simulink model "VirtualADAPT"  (Simulink 8.9)
settings: Solver=ode45  StartTime=0.0  StopTime=inf  RelTol=1e-3  EnableMultiTasking=on
PostLoadFcn:
  | FaultInjectionGUIHandle = FaultInjectionGUI;
InitFcn:
  | VirtualADAPTDataStructure
undefined symbols (not defined in the file or in its folder): ln
libraries: simulink x363
(part 25 of 26)

== system VirtualADAPT/VirtualADAPTv1/PowerDistribution/ACLoadBank1/L1A/LGT6/LightBulb2/R/Computing R ==
  In1 : Inport
  RNominal : Inport  Port=2
  Fault Profile : Inport  Port=3
  Step : Inport  Port=4
  Ramp : Inport  Port=5
  JuncDB : Inport  Port=6
  Bond : Inport  Port=7
  Junction Type : Inport  Port=8
  CalcCausality : Fcn  Expr=(u(1) == u(2) && u(3) ==1) || (u(1) !=u(2) && u(3) == 0)
  CalcOutput : Fcn  Expr=(u(1)*u(2)^u(3))
  MinusOne : Constant  Value=-1
  Mux1 : Mux  Inputs=3
  Mux2 : Mux  Inputs=3
  One : Constant
  Switch1 : MultiPortSwitch  SaturateOnIntegerOverflow=on  InputSameDT=on
  Switch2 : MultiPortSwitch  DataPortOrder=Zero-based contiguous  Inputs=2  zeroidx=on  SaturateOnIntegerOverflow=on  InputSameDT=on
  Out1 : Outport
  Out2 : Outport  Port=2
  wires:
  In1/1 -> Mux2/1
  Fault Profile/1 -> Switch1/1
  RNominal/1 -> Switch1/2
  Step/1 -> Switch1/3
  Ramp/1 -> Switch1/4
  Switch1/1 -> Out2/1, Mux2/2
  JuncDB/1 -> Mux1/1
  Bond/1 -> Mux1/2
  Junction Type/1 -> Mux1/3
  Mux1/1 -> CalcCausality/1
  CalcCausality/1 -> Switch2/1
  One/1 -> Switch2/2
  MinusOne/1 -> Switch2/3
  Switch2/1 -> Mux2/3
  Mux2/1 -> CalcOutput/1
  CalcOutput/1 -> Out1/1

== system VirtualADAPT/VirtualADAPTv1/PowerDistribution/ACLoadBank1/L1A/LGT6/LightBulb2/TemperatureModel/AmbTemp ==
  F : Inport
  Se : Inport  Port=2
  Bias : Step  Time=FT  After=FM  SampleTime=0
  Fault Inject : Subsystem
  Fault Profile : Constant  Value=FP
  Mult2 : Product  SaturateOnIntegerOverflow=on  RndMeth=Zero  InputSameDT=on  OutDataTypeStr=Inherit: Same as first input
  Multiply : Product  SaturateOnIntegerOverflow=on  RndMeth=Zero  InputSameDT=on  OutDataTypeStr=Inherit: Same as first input
  Ramp : simulink/Sources/Ramp  slope=FM  start=FT  X0=0
  Step : Step  Time=FT  Before=1  After=(1 + FM)  SampleTime=0
  StuckAt : Step  Time=FT  Before=1  After=0  SampleTime=0
  StuckAt2 : Step  Time=FT  After=FM  SampleTime=0
  Sum : Sum  SaturateOnIntegerOverflow=on  InputSameDT=on  OutDataTypeStr=Inherit: Same as first input
  Sum2 : Sum  SaturateOnIntegerOverflow=on  InputSameDT=on  OutDataTypeStr=Inherit: Same as first input
  Sum3 : Sum  SaturateOnIntegerOverflow=on  InputSameDT=on  OutDataTypeStr=Inherit: Same as first input
  E : Outport
  wires:
  Fault Inject/1(Out1) -> E/1
  Se/1 -> Fault Inject/1(Nominal), Multiply/1, Sum/1, Sum2/1, Mult2/1
  Fault Profile/1 -> Fault Inject/2(Fault Profile)
  Step/1 -> Multiply/2
  Ramp/1 -> Sum/2
  Multiply/1 -> Fault Inject/3(Step)
  Sum/1 -> Fault Inject/4(Ramp)
  Bias/1 -> Sum2/2
  Sum2/1 -> Fault Inject/5(Bias)
  StuckAt/1 -> Mult2/2
  Mult2/1 -> Sum3/1
  StuckAt2/1 -> Sum3/2
  Sum3/1 -> Fault Inject/6(stuckAt)

== system VirtualADAPT/VirtualADAPTv1/PowerDistribution/ACLoadBank1/L1A/LGT6/LightBulb2/TemperatureModel/Ctheta ==
  F : Inport
  Capacitance : Constant  Value=C
  Data Type Conversion : DataTypeConversion  OutDataTypeStr=boolean
  Detect Change : simulink/Logic and Bit Operations/Detect Change  vinit=0  InputProcessing=Inherited  OutDataTypeStr=uint8
  Fault Inject : Subsystem
  Fault Profile : Constant  Value=FP
  InitialCondition : Constant  Value=IC
  Integrator : Integrator  ExternalReset=either  InitialConditionSource=external
  Product1 : Product  SaturateOnIntegerOverflow=on  RndMeth=Zero  InputSameDT=on  OutDataTypeStr=Inherit: Same as first input
  Product2 : Product  SaturateOnIntegerOverflow=on  RndMeth=Zero  InputSameDT=on  OutDataTypeStr=Inherit: Same as first input
  Ramp : simulink/Sources/Ramp  slope=FM  start=FT  X0=C
  Reciprocal : Math  Operator=reciprocal  SaturateOnIntegerOverflow=on
  Step : Step  Time=FT  Before=C  After=(1 + FM)*C  SampleTime=0
  E : Outport
  C : Outport  Port=2
  q : Outport  Port=3
  wires:
  F/1 -> Integrator/1
  InitialCondition/1 -> Product1/1, Detect Change/1
  Capacitance/1 -> Fault Inject/1(Nominal), Product1/2
  Product1/1 -> Integrator/3
  Integrator/1 -> Product2/1, q/1
  Fault Inject/1(Out1) -> Reciprocal/1, C/1
  Reciprocal/1 -> Product2/2
  Fault Profile/1 -> Fault Inject/2(Fault Profile)
  Step/1 -> Fault Inject/3(Step)
  Ramp/1 -> Fault Inject/4(Ramp)
  Product2/1 -> E/1
  Detect Change/1 -> Data Type Conversion/1
  Data Type Conversion/1 -> Integrator/2

== system VirtualADAPT/VirtualADAPTv1/PowerDistribution/ACLoadBank1/L1A/LGT6/LightBulb2/TemperatureModel/ModAmbTemp ==
  u(1) : Inport
  Function : Fcn  Expr=-u(1)
  Mux : Mux  Inputs=1
  y(1) : Outport
  wires:
  u(1)/1 -> Mux/1
  Mux/1 -> Function/1
  Function/1 -> y(1)/1

== system VirtualADAPT/VirtualADAPTv1/PowerDistribution/ACLoadBank1/L1A/LGT6/LightBulb2/TemperatureModel/ModPs ==
  u(1) : Inport
  Function : Fcn  Expr=u(1)
  Mux : Mux  Inputs=1
  y(1) : Outport
  wires:
  u(1)/1 -> Mux/1
  Mux/1 -> Function/1
  Function/1 -> y(1)/1

== system VirtualADAPT/VirtualADAPTv1/PowerDistribution/ACLoadBank1/L1A/LGT6/LightBulb2/TemperatureModel/OJ ==
  u(1) : Inport
  u(2) : Inport  Port=2
  u(3) : Inport  Port=3
  Adder : Sum  SaturateOnIntegerOverflow=on  InputSameDT=on  OutDataTypeStr=Inherit: Same as first input
  Gain : Gain  SaturateOnIntegerOverflow=on  OutDataTypeStr=Inherit: Same as input  ParamDataTypeStr=Inherit: Same as input
  y(1) : Outport
  y(2) : Outport  Port=2
  y(3) : Outport  Port=3
  O : Outport  Port=4
  wires:
  Adder/1 -> Gain/1
  u(2)/1 -> y(1)/1, y(3)/1, O/1
  u(1)/1 -> Adder/1
  Gain/1 -> y(2)/1
  u(3)/1 -> Adder/2

== system VirtualADAPT/VirtualADAPTv1/PowerDistribution/ACLoadBank1/L1A/LGT6/LightBulb2/TemperatureModel/Rtheta ==
  In : Inport
  Bond : Constant  Value=2
  Computing R : Subsystem
  Fault Profile : Constant  Value=FP
  JuncDB : Constant  Value=2
  Junction Type : Constant
  Ramp : simulink/Sources/Ramp  slope=FM  start=FT  X0=R
  Resistor Value : Constant  Value=R
  Step : Step  Time=FT  Before=R  After=(1 + FM)*R  SampleTime=0
  Out : Outport
  R : Outport  Port=2
  wires:
  Computing R/1(Out1) -> Out/1
  In/1 -> Computing R/1(In1)
  Resistor Value/1 -> Computing R/2(RNominal)
  Fault Profile/1 -> Computing R/3(Fault Profile)
  Step/1 -> Computing R/4(Step)
  Ramp/1 -> Computing R/5(Ramp)
  JuncDB/1 -> Computing R/6(JuncDB)
  Bond/1 -> Computing R/7(Bond)
  Junction Type/1 -> Computing R/8(Junction Type)
  Computing R/2(Out2) -> R/1

== system VirtualADAPT/VirtualADAPTv1/PowerDistribution/ACLoadBank1/L1A/LGT6/LightBulb2/TemperatureModel/SfPs ==
  E : Inport
  Sf : Inport  Port=2
  Bias : Step  Time=FT  After=FM  SampleTime=0
  Fault Inject : Subsystem
  Fault Profile : Constant  Value=FP
  Mult2 : Product  SaturateOnIntegerOverflow=on  RndMeth=Zero  InputSameDT=on  OutDataTypeStr=Inherit: Same as first input
  Multiply : Product  SaturateOnIntegerOverflow=on  RndMeth=Zero  InputSameDT=on  OutDataTypeStr=Inherit: Same as first input
  Ramp : simulink/Sources/Ramp  slope=FM  start=FT  X0=0
  Step : Step  Time=FT  Before=1  After=(1 + FM)  SampleTime=0
  StuckAt : Step  Time=FT  Before=1  After=0  SampleTime=0
  StuckAt2 : Step  Time=FT  After=FM  SampleTime=0
  Sum : Sum  SaturateOnIntegerOverflow=on  InputSameDT=on  OutDataTypeStr=Inherit: Same as first input
  Sum2 : Sum  SaturateOnIntegerOverflow=on  InputSameDT=on  OutDataTypeStr=Inherit: Same as first input
  Sum3 : Sum  SaturateOnIntegerOverflow=on  InputSameDT=on  OutDataTypeStr=Inherit: Same as first input
  F : Outport
  wires:
  Fault Inject/1(Out1) -> F/1
  Sf/1 -> Fault Inject/1(Nominal), Multiply/1, Sum/1, Sum2/1, Mult2/1
  Fault Profile/1 -> Fault Inject/2(Fault Profile)
  Step/1 -> Multiply/2
  Ramp/1 -> Sum/2
  Multiply/1 -> Fault Inject/3(Step)
  Sum/1 -> Fault Inject/4(Ramp)
  Bias/1 -> Sum2/2
  Sum2/1 -> Fault Inject/5(Bias)
  StuckAt/1 -> Mult2/2
  Mult2/1 -> Sum3/1
  StuckAt2/1 -> Sum3/2
  Sum3/1 -> Fault Inject/6(stuckAt)

== system VirtualADAPT/VirtualADAPTv1/PowerDistribution/ACLoadBank1/L1A/LGT6/LightBulb2/TemperatureModel/theta ==
  u(1) : Inport
  u(2) : Inport  Port=2
  u(3) : Inport  Port=3
  Adder : Sum  Inputs=+-  SaturateOnIntegerOverflow=on  InputSameDT=on  OutDataTypeStr=Inherit: Same as first input
  Gain : Gain  SaturateOnIntegerOverflow=on  OutDataTypeStr=Inherit: Same as input  ParamDataTypeStr=Inherit: Same as input
  y(1) : Outport
  y(2) : Outport  Port=2
  y(3) : Outport  Port=3
  O : Outport  Port=4
  wires:
  Adder/1 -> Gain/1
  u(3)/1 -> y(1)/1, y(2)/1, O/1
  u(1)/1 -> Adder/1
  u(2)/1 -> Adder/2
  Gain/1 -> y(3)/1

== system VirtualADAPT/VirtualADAPTv1/PowerDistribution/ACLoadBank1/L1A/LGT6/LightBulb3/R/Computing R ==
  In1 : Inport
  RNominal : Inport  Port=2
  Fault Profile : Inport  Port=3
  Step : Inport  Port=4
  Ramp : Inport  Port=5
  JuncDB : Inport  Port=6
  Bond : Inport  Port=7
  Junction Type : Inport  Port=8
  CalcCausality : Fcn  Expr=(u(1) == u(2) && u(3) ==1) || (u(1) !=u(2) && u(3) == 0)
  CalcOutput : Fcn  Expr=(u(1)*u(2)^u(3))
  MinusOne : Constant  Value=-1
  Mux1 : Mux  Inputs=3
  Mux2 : Mux  Inputs=3
  One : Constant
  Switch1 : MultiPortSwitch  SaturateOnIntegerOverflow=on  InputSameDT=on
  Switch2 : MultiPortSwitch  DataPortOrder=Zero-based contiguous  Inputs=2  zeroidx=on  SaturateOnIntegerOverflow=on  InputSameDT=on
  Out1 : Outport
  Out2 : Outport  Port=2
  wires:
  In1/1 -> Mux2/1
  Fault Profile/1 -> Switch1/1
  RNominal/1 -> Switch1/2
  Step/1 -> Switch1/3
  Ramp/1 -> Switch1/4
  Switch1/1 -> Out2/1, Mux2/2
  JuncDB/1 -> Mux1/1
  Bond/1 -> Mux1/2
  Junction Type/1 -> Mux1/3
  Mux1/1 -> CalcCausality/1
  CalcCausality/1 -> Switch2/1
  One/1 -> Switch2/2
  MinusOne/1 -> Switch2/3
  Switch2/1 -> Mux2/3
  Mux2/1 -> CalcOutput/1
  CalcOutput/1 -> Out1/1

== system VirtualADAPT/VirtualADAPTv1/PowerDistribution/ACLoadBank1/L1A/LGT6/LightBulb3/TemperatureModel/AmbTemp ==
  F : Inport
  Se : Inport  Port=2
  Bias : Step  Time=FT  After=FM  SampleTime=0
  Fault Inject : Subsystem
  Fault Profile : Constant  Value=FP
  Mult2 : Product  SaturateOnIntegerOverflow=on  RndMeth=Zero  InputSameDT=on  OutDataTypeStr=Inherit: Same as first input
  Multiply : Product  SaturateOnIntegerOverflow=on  RndMeth=Zero  InputSameDT=on  OutDataTypeStr=Inherit: Same as first input
  Ramp : simulink/Sources/Ramp  slope=FM  start=FT  X0=0
  Step : Step  Time=FT  Before=1  After=(1 + FM)  SampleTime=0
  StuckAt : Step  Time=FT  Before=1  After=0  SampleTime=0
  StuckAt2 : Step  Time=FT  After=FM  SampleTime=0
  Sum : Sum  SaturateOnIntegerOverflow=on  InputSameDT=on  OutDataTypeStr=Inherit: Same as first input
  Sum2 : Sum  SaturateOnIntegerOverflow=on  InputSameDT=on  OutDataTypeStr=Inherit: Same as first input
  Sum3 : Sum  SaturateOnIntegerOverflow=on  InputSameDT=on  OutDataTypeStr=Inherit: Same as first input
  E : Outport
  wires:
  Fault Inject/1(Out1) -> E/1
  Se/1 -> Fault Inject/1(Nominal), Multiply/1, Sum/1, Sum2/1, Mult2/1
  Fault Profile/1 -> Fault Inject/2(Fault Profile)
  Step/1 -> Multiply/2
  Ramp/1 -> Sum/2
  Multiply/1 -> Fault Inject/3(Step)
  Sum/1 -> Fault Inject/4(Ramp)
  Bias/1 -> Sum2/2
  Sum2/1 -> Fault Inject/5(Bias)
  StuckAt/1 -> Mult2/2
  Mult2/1 -> Sum3/1
  StuckAt2/1 -> Sum3/2
  Sum3/1 -> Fault Inject/6(stuckAt)

== system VirtualADAPT/VirtualADAPTv1/PowerDistribution/ACLoadBank1/L1A/LGT6/LightBulb3/TemperatureModel/Ctheta ==
  F : Inport
  Capacitance : Constant  Value=C
  Data Type Conversion : DataTypeConversion  OutDataTypeStr=boolean
  Detect Change : simulink/Logic and Bit Operations/Detect Change  vinit=0  InputProcessing=Inherited  OutDataTypeStr=uint8
  Fault Inject : Subsystem
  Fault Profile : Constant  Value=FP
  InitialCondition : Constant  Value=IC
  Integrator : Integrator  ExternalReset=either  InitialConditionSource=external
  Product1 : Product  SaturateOnIntegerOverflow=on  RndMeth=Zero  InputSameDT=on  OutDataTypeStr=Inherit: Same as first input
  Product2 : Product  SaturateOnIntegerOverflow=on  RndMeth=Zero  InputSameDT=on  OutDataTypeStr=Inherit: Same as first input
  Ramp : simulink/Sources/Ramp  slope=FM  start=FT  X0=C
  Reciprocal : Math  Operator=reciprocal  SaturateOnIntegerOverflow=on
  Step : Step  Time=FT  Before=C  After=(1 + FM)*C  SampleTime=0
  E : Outport
  C : Outport  Port=2
  q : Outport  Port=3
  wires:
  F/1 -> Integrator/1
  InitialCondition/1 -> Product1/1, Detect Change/1
  Capacitance/1 -> Fault Inject/1(Nominal), Product1/2
  Product1/1 -> Integrator/3
  Integrator/1 -> Product2/1, q/1
  Fault Inject/1(Out1) -> Reciprocal/1, C/1
  Reciprocal/1 -> Product2/2
  Fault Profile/1 -> Fault Inject/2(Fault Profile)
  Step/1 -> Fault Inject/3(Step)
  Ramp/1 -> Fault Inject/4(Ramp)
  Product2/1 -> E/1
  Detect Change/1 -> Data Type Conversion/1
  Data Type Conversion/1 -> Integrator/2

== system VirtualADAPT/VirtualADAPTv1/PowerDistribution/ACLoadBank1/L1A/LGT6/LightBulb3/TemperatureModel/ModAmbTemp ==
  u(1) : Inport
  Function : Fcn  Expr=-u(1)
  Mux : Mux  Inputs=1
  y(1) : Outport
  wires:
  u(1)/1 -> Mux/1
  Mux/1 -> Function/1
  Function/1 -> y(1)/1

== system VirtualADAPT/VirtualADAPTv1/PowerDistribution/ACLoadBank1/L1A/LGT6/LightBulb3/TemperatureModel/ModPs ==
  u(1) : Inport
  Function : Fcn  Expr=u(1)
  Mux : Mux  Inputs=1
  y(1) : Outport
  wires:
  u(1)/1 -> Mux/1
  Mux/1 -> Function/1
  Function/1 -> y(1)/1

== system VirtualADAPT/VirtualADAPTv1/PowerDistribution/ACLoadBank1/L1A/LGT6/LightBulb3/TemperatureModel/OJ ==
  u(1) : Inport
  u(2) : Inport  Port=2
  u(3) : Inport  Port=3
  Adder : Sum  SaturateOnIntegerOverflow=on  InputSameDT=on  OutDataTypeStr=Inherit: Same as first input
  Gain : Gain  SaturateOnIntegerOverflow=on  OutDataTypeStr=Inherit: Same as input  ParamDataTypeStr=Inherit: Same as input
  y(1) : Outport
  y(2) : Outport  Port=2
  y(3) : Outport  Port=3
  O : Outport  Port=4
  wires:
  Adder/1 -> Gain/1
  u(3)/1 -> y(1)/1, y(2)/1, O/1
  u(1)/1 -> Adder/1
  u(2)/1 -> Adder/2
  Gain/1 -> y(3)/1

== system VirtualADAPT/VirtualADAPTv1/PowerDistribution/ACLoadBank1/L1A/LGT6/LightBulb3/TemperatureModel/Rtheta ==
  In : Inport
  Bond : Constant  Value=3
  Computing R : Subsystem
  Fault Profile : Constant  Value=FP
  JuncDB : Constant  Value=3
  Junction Type : Constant
  Ramp : simulink/Sources/Ramp  slope=FM  start=FT  X0=R
  Resistor Value : Constant  Value=R
  Step : Step  Time=FT  Before=R  After=(1 + FM)*R  SampleTime=0
  Out : Outport
  R : Outport  Port=2
  wires:
  Computing R/1(Out1) -> Out/1
  In/1 -> Computing R/1(In1)
  Resistor Value/1 -> Computing R/2(RNominal)
  Fault Profile/1 -> Computing R/3(Fault Profile)
  Step/1 -> Computing R/4(Step)
  Ramp/1 -> Computing R/5(Ramp)
  JuncDB/1 -> Computing R/6(JuncDB)
  Bond/1 -> Computing R/7(Bond)
  Junction Type/1 -> Computing R/8(Junction Type)
  Computing R/2(Out2) -> R/1

== system VirtualADAPT/VirtualADAPTv1/PowerDistribution/ACLoadBank1/L1A/LGT6/LightBulb3/TemperatureModel/SfPs ==
  E : Inport
  Sf : Inport  Port=2
  Bias : Step  Time=FT  After=FM  SampleTime=0
  Fault Inject : Subsystem
  Fault Profile : Constant  Value=FP
  Mult2 : Product  SaturateOnIntegerOverflow=on  RndMeth=Zero  InputSameDT=on  OutDataTypeStr=Inherit: Same as first input
  Multiply : Product  SaturateOnIntegerOverflow=on  RndMeth=Zero  InputSameDT=on  OutDataTypeStr=Inherit: Same as first input
  Ramp : simulink/Sources/Ramp  slope=FM  start=FT  X0=0
  Step : Step  Time=FT  Before=1  After=(1 + FM)  SampleTime=0
  StuckAt : Step  Time=FT  Before=1  After=0  SampleTime=0
  StuckAt2 : Step  Time=FT  After=FM  SampleTime=0
  Sum : Sum  SaturateOnIntegerOverflow=on  InputSameDT=on  OutDataTypeStr=Inherit: Same as first input
  Sum2 : Sum  SaturateOnIntegerOverflow=on  InputSameDT=on  OutDataTypeStr=Inherit: Same as first input
  Sum3 : Sum  SaturateOnIntegerOverflow=on  InputSameDT=on  OutDataTypeStr=Inherit: Same as first input
  F : Outport
  wires:
  Fault Inject/1(Out1) -> F/1
  Sf/1 -> Fault Inject/1(Nominal), Multiply/1, Sum/1, Sum2/1, Mult2/1
  Fault Profile/1 -> Fault Inject/2(Fault Profile)
  Step/1 -> Multiply/2
  Ramp/1 -> Sum/2
  Multiply/1 -> Fault Inject/3(Step)
  Sum/1 -> Fault Inject/4(Ramp)
  Bias/1 -> Sum2/2
  Sum2/1 -> Fault Inject/5(Bias)
  StuckAt/1 -> Mult2/2
  Mult2/1 -> Sum3/1
  StuckAt2/1 -> Sum3/2
  Sum3/1 -> Fault Inject/6(stuckAt)

== system VirtualADAPT/VirtualADAPTv1/PowerDistribution/ACLoadBank1/L1A/LGT6/LightBulb3/TemperatureModel/theta ==
  u(1) : Inport
  u(2) : Inport  Port=2
  u(3) : Inport  Port=3
  Adder : Sum  Inputs=-+  SaturateOnIntegerOverflow=on  InputSameDT=on  OutDataTypeStr=Inherit: Same as first input
  Gain : Gain  SaturateOnIntegerOverflow=on  OutDataTypeStr=Inherit: Same as input  ParamDataTypeStr=Inherit: Same as input
  y(1) : Outport
  y(2) : Outport  Port=2
  y(3) : Outport  Port=3
  O : Outport  Port=4
  wires:
  Adder/1 -> Gain/1
  Gain/1 -> y(1)/1
  u(1)/1 -> y(2)/1, y(3)/1, O/1
  u(2)/1 -> Adder/1
  u(3)/1 -> Adder/2

== system VirtualADAPT/VirtualADAPTv1/PowerDistribution/ACLoadBank1/L1F/LGT4/TemperatureModel/AmbTemp/Fault Inject ==
  Nominal : Inport
  Fault Profile : Inport  Port=2
  Step : Inport  Port=3
  Ramp : Inport  Port=4
  Bias : Inport  Port=5
  stuckAt : Inport  Port=6
  Switch1 : MultiPortSwitch  Inputs=5  SaturateOnIntegerOverflow=on  InputSameDT=on
  Out1 : Outport
  wires:
  Nominal/1 -> Switch1/2
  Fault Profile/1 -> Switch1/1
  Step/1 -> Switch1/3
  Ramp/1 -> Switch1/4
  Bias/1 -> Switch1/5
  stuckAt/1 -> Switch1/6
  Switch1/1 -> Out1/1

== system VirtualADAPT/VirtualADAPTv1/PowerDistribution/ACLoadBank1/L1F/LGT4/TemperatureModel/Ctheta/Fault Inject ==
  Nominal : Inport
  Fault Profile : Inport  Port=2
  Step : Inport  Port=3
  Ramp : Inport  Port=4
  Bias : Inport  Port=5
  stuckAt : Inport  Port=6
  Switch1 : MultiPortSwitch  Inputs=5  SaturateOnIntegerOverflow=on  InputSameDT=on
  Out1 : Outport
  wires:
  Nominal/1 -> Switch1/2
  Fault Profile/1 -> Switch1/1
  Step/1 -> Switch1/3
  Ramp/1 -> Switch1/4
  Bias/1 -> Switch1/5
  stuckAt/1 -> Switch1/6
  Switch1/1 -> Out1/1

== system VirtualADAPT/VirtualADAPTv1/PowerDistribution/ACLoadBank1/L1F/LGT4/TemperatureModel/Rtheta/Computing R ==
  In1 : Inport
  RNominal : Inport  Port=2
  Fault Profile : Inport  Port=3
  Step : Inport  Port=4
  Ramp : Inport  Port=5
  JuncDB : Inport  Port=6
  Bond : Inport  Port=7
  Junction Type : Inport  Port=8
  CalcCausality : Fcn  Expr=(u(1) == u(2) && u(3) ==1) || (u(1) !=u(2) && u(3) == 0)
  CalcOutput : Fcn  Expr=(u(1)*u(2)^u(3))
  MinusOne : Constant  Value=-1
  Mux1 : Mux  Inputs=3
  Mux2 : Mux  Inputs=3
  One : Constant
  Switch1 : MultiPortSwitch  SaturateOnIntegerOverflow=on  InputSameDT=on
  Switch2 : MultiPortSwitch  DataPortOrder=Zero-based contiguous  Inputs=2  zeroidx=on  SaturateOnIntegerOverflow=on  InputSameDT=on
  Out1 : Outport
  Out2 : Outport  Port=2
  wires:
  In1/1 -> Mux2/1
  Fault Profile/1 -> Switch1/1
  RNominal/1 -> Switch1/2
  Step/1 -> Switch1/3
  Ramp/1 -> Switch1/4
  Switch1/1 -> Out2/1, Mux2/2
  JuncDB/1 -> Mux1/1
  Bond/1 -> Mux1/2
  Junction Type/1 -> Mux1/3
  Mux1/1 -> CalcCausality/1
  CalcCausality/1 -> Switch2/1
  One/1 -> Switch2/2
  MinusOne/1 -> Switch2/3
  Switch2/1 -> Mux2/3
  Mux2/1 -> CalcOutput/1
  CalcOutput/1 -> Out1/1

== system VirtualADAPT/VirtualADAPTv1/PowerDistribution/ACLoadBank1/L1F/LGT4/TemperatureModel/SfPs/Fault Inject ==
  Nominal : Inport
  Fault Profile : Inport  Port=2
  Step : Inport  Port=3
  Ramp : Inport  Port=4
  Bias : Inport  Port=5
  stuckAt : Inport  Port=6
  Switch1 : MultiPortSwitch  Inputs=5  SaturateOnIntegerOverflow=on  InputSameDT=on
  Out1 : Outport
  wires:
  Nominal/1 -> Switch1/2
  Fault Profile/1 -> Switch1/1
  Step/1 -> Switch1/3
  Ramp/1 -> Switch1/4
  Bias/1 -> Switch1/5
  stuckAt/1 -> Switch1/6
  Switch1/1 -> Out1/1

== system VirtualADAPT/VirtualADAPTv1/PowerDistribution/ACLoadBank2/L2B/LGT2/TemperatureModel/AmbTemp/Fault Inject ==
  Nominal : Inport
  Fault Profile : Inport  Port=2
  Step : Inport  Port=3
  Ramp : Inport  Port=4
  Bias : Inport  Port=5
  stuckAt : Inport  Port=6
  Switch1 : MultiPortSwitch  Inputs=5  SaturateOnIntegerOverflow=on  InputSameDT=on
  Out1 : Outport
  wires:
  Nominal/1 -> Switch1/2
  Fault Profile/1 -> Switch1/1
  Step/1 -> Switch1/3
  Ramp/1 -> Switch1/4
  Bias/1 -> Switch1/5
  stuckAt/1 -> Switch1/6
  Switch1/1 -> Out1/1

== system VirtualADAPT/VirtualADAPTv1/PowerDistribution/ACLoadBank2/L2B/LGT2/TemperatureModel/Ctheta/Fault Inject ==
  Nominal : Inport
  Fault Profile : Inport  Port=2
  Step : Inport  Port=3
  Ramp : Inport  Port=4
  Bias : Inport  Port=5
  stuckAt : Inport  Port=6
  Switch1 : MultiPortSwitch  Inputs=5  SaturateOnIntegerOverflow=on  InputSameDT=on
  Out1 : Outport
  wires:
  Nominal/1 -> Switch1/2
  Fault Profile/1 -> Switch1/1
  Step/1 -> Switch1/3
  Ramp/1 -> Switch1/4
  Bias/1 -> Switch1/5
  stuckAt/1 -> Switch1/6
  Switch1/1 -> Out1/1

== system VirtualADAPT/VirtualADAPTv1/PowerDistribution/ACLoadBank2/L2B/LGT2/TemperatureModel/Rtheta/Computing R ==
  In1 : Inport
  RNominal : Inport  Port=2
  Fault Profile : Inport  Port=3
  Step : Inport  Port=4
  Ramp : Inport  Port=5
  JuncDB : Inport  Port=6
  Bond : Inport  Port=7
  Junction Type : Inport  Port=8
  CalcCausality : Fcn  Expr=(u(1) == u(2) && u(3) ==1) || (u(1) !=u(2) && u(3) == 0)
  CalcOutput : Fcn  Expr=(u(1)*u(2)^u(3))
  MinusOne : Constant  Value=-1
  Mux1 : Mux  Inputs=3
  Mux2 : Mux  Inputs=3
  One : Constant
  Switch1 : MultiPortSwitch  SaturateOnIntegerOverflow=on  InputSameDT=on
  Switch2 : MultiPortSwitch  DataPortOrder=Zero-based contiguous  Inputs=2  zeroidx=on  SaturateOnIntegerOverflow=on  InputSameDT=on
  Out1 : Outport
  Out2 : Outport  Port=2
  wires:
  In1/1 -> Mux2/1
  Fault Profile/1 -> Switch1/1
  RNominal/1 -> Switch1/2
  Step/1 -> Switch1/3
  Ramp/1 -> Switch1/4
  Switch1/1 -> Out2/1, Mux2/2
  JuncDB/1 -> Mux1/1
  Bond/1 -> Mux1/2
  Junction Type/1 -> Mux1/3
  Mux1/1 -> CalcCausality/1
  CalcCausality/1 -> Switch2/1
  One/1 -> Switch2/2
  MinusOne/1 -> Switch2/3
  Switch2/1 -> Mux2/3
  Mux2/1 -> CalcOutput/1
  CalcOutput/1 -> Out1/1

== system VirtualADAPT/VirtualADAPTv1/PowerDistribution/ACLoadBank2/L2B/LGT2/TemperatureModel/SfPs/Fault Inject ==
  Nominal : Inport
  Fault Profile : Inport  Port=2
  Step : Inport  Port=3
  Ramp : Inport  Port=4
  Bias : Inport  Port=5
  stuckAt : Inport  Port=6
  Switch1 : MultiPortSwitch  Inputs=5  SaturateOnIntegerOverflow=on  InputSameDT=on
  Out1 : Outport
  wires:
  Nominal/1 -> Switch1/2
  Fault Profile/1 -> Switch1/1
  Step/1 -> Switch1/3
  Ramp/1 -> Switch1/4
  Bias/1 -> Switch1/5
  stuckAt/1 -> Switch1/6
  Switch1/1 -> Out1/1

== system VirtualADAPT/VirtualADAPTv1/PowerDistribution/ACLoadBank2/L2E/LGT7/LightBulb1/R/Computing R ==
  In1 : Inport
  RNominal : Inport  Port=2
  Fault Profile : Inport  Port=3
  Step : Inport  Port=4
  Ramp : Inport  Port=5
  JuncDB : Inport  Port=6
  Bond : Inport  Port=7
  Junction Type : Inport  Port=8
  CalcCausality : Fcn  Expr=(u(1) == u(2) && u(3) ==1) || (u(1) !=u(2) && u(3) == 0)
  CalcOutput : Fcn  Expr=(u(1)*u(2)^u(3))
  MinusOne : Constant  Value=-1
  Mux1 : Mux  Inputs=3
  Mux2 : Mux  Inputs=3
  One : Constant
  Switch1 : MultiPortSwitch  SaturateOnIntegerOverflow=on  InputSameDT=on
  Switch2 : MultiPortSwitch  DataPortOrder=Zero-based contiguous  Inputs=2  zeroidx=on  SaturateOnIntegerOverflow=on  InputSameDT=on
  Out1 : Outport
  Out2 : Outport  Port=2
  wires:
  In1/1 -> Mux2/1
  Fault Profile/1 -> Switch1/1
  RNominal/1 -> Switch1/2
  Step/1 -> Switch1/3
  Ramp/1 -> Switch1/4
  Switch1/1 -> Out2/1, Mux2/2
  JuncDB/1 -> Mux1/1
  Bond/1 -> Mux1/2
  Junction Type/1 -> Mux1/3
  Mux1/1 -> CalcCausality/1
  CalcCausality/1 -> Switch2/1
  One/1 -> Switch2/2
  MinusOne/1 -> Switch2/3
  Switch2/1 -> Mux2/3
  Mux2/1 -> CalcOutput/1
  CalcOutput/1 -> Out1/1

== system VirtualADAPT/VirtualADAPTv1/PowerDistribution/ACLoadBank2/L2E/LGT7/LightBulb1/TemperatureModel/AmbTemp ==
  F : Inport
  Se : Inport  Port=2
  Bias : Step  Time=FT  After=FM  SampleTime=0
  Fault Inject : Subsystem
  Fault Profile : Constant  Value=FP
  Mult2 : Product  SaturateOnIntegerOverflow=on  RndMeth=Zero  InputSameDT=on  OutDataTypeStr=Inherit: Same as first input
  Multiply : Product  SaturateOnIntegerOverflow=on  RndMeth=Zero  InputSameDT=on  OutDataTypeStr=Inherit: Same as first input
  Ramp : simulink/Sources/Ramp  slope=FM  start=FT  X0=0
  Step : Step  Time=FT  Before=1  After=(1 + FM)  SampleTime=0
  StuckAt : Step  Time=FT  Before=1  After=0  SampleTime=0
  StuckAt2 : Step  Time=FT  After=FM  SampleTime=0
  Sum : Sum  SaturateOnIntegerOverflow=on  InputSameDT=on  OutDataTypeStr=Inherit: Same as first input
  Sum2 : Sum  SaturateOnIntegerOverflow=on  InputSameDT=on  OutDataTypeStr=Inherit: Same as first input
  Sum3 : Sum  SaturateOnIntegerOverflow=on  InputSameDT=on  OutDataTypeStr=Inherit: Same as first input
  E : Outport
  wires:
  Fault Inject/1(Out1) -> E/1
  Se/1 -> Fault Inject/1(Nominal), Multiply/1, Sum/1, Sum2/1, Mult2/1
  Fault Profile/1 -> Fault Inject/2(Fault Profile)
  Step/1 -> Multiply/2
  Ramp/1 -> Sum/2
  Multiply/1 -> Fault Inject/3(Step)
  Sum/1 -> Fault Inject/4(Ramp)
  Bias/1 -> Sum2/2
  Sum2/1 -> Fault Inject/5(Bias)
  StuckAt/1 -> Mult2/2
  Mult2/1 -> Sum3/1
  StuckAt2/1 -> Sum3/2
  Sum3/1 -> Fault Inject/6(stuckAt)

== system VirtualADAPT/VirtualADAPTv1/PowerDistribution/ACLoadBank2/L2E/LGT7/LightBulb1/TemperatureModel/Ctheta ==
  F : Inport
  Capacitance : Constant  Value=C
  Data Type Conversion : DataTypeConversion  OutDataTypeStr=boolean
  Detect Change : simulink/Logic and Bit Operations/Detect Change  vinit=0  InputProcessing=Inherited  OutDataTypeStr=uint8
  Fault Inject : Subsystem
  Fault Profile : Constant  Value=FP
  InitialCondition : Constant  Value=IC
  Integrator : Integrator  ExternalReset=either  InitialConditionSource=external
  Product1 : Product  SaturateOnIntegerOverflow=on  RndMeth=Zero  InputSameDT=on  OutDataTypeStr=Inherit: Same as first input
  Product2 : Product  SaturateOnIntegerOverflow=on  RndMeth=Zero  InputSameDT=on  OutDataTypeStr=Inherit: Same as first input
  Ramp : simulink/Sources/Ramp  slope=FM  start=FT  X0=C
  Reciprocal : Math  Operator=reciprocal  SaturateOnIntegerOverflow=on
  Step : Step  Time=FT  Before=C  After=(1 + FM)*C  SampleTime=0
  E : Outport
  C : Outport  Port=2
  q : Outport  Port=3
  wires:
  F/1 -> Integrator/1
  InitialCondition/1 -> Product1/1, Detect Change/1
  Capacitance/1 -> Fault Inject/1(Nominal), Product1/2
  Product1/1 -> Integrator/3
  Integrator/1 -> Product2/1, q/1
  Fault Inject/1(Out1) -> Reciprocal/1, C/1
  Reciprocal/1 -> Product2/2
  Fault Profile/1 -> Fault Inject/2(Fault Profile)
  Step/1 -> Fault Inject/3(Step)
  Ramp/1 -> Fault Inject/4(Ramp)
  Product2/1 -> E/1
  Detect Change/1 -> Data Type Conversion/1
  Data Type Conversion/1 -> Integrator/2

== system VirtualADAPT/VirtualADAPTv1/PowerDistribution/ACLoadBank2/L2E/LGT7/LightBulb1/TemperatureModel/ModAmbTemp ==
  u(1) : Inport
  Function : Fcn  Expr=-u(1)
  Mux : Mux  Inputs=1
  y(1) : Outport
  wires:
  u(1)/1 -> Mux/1
  Mux/1 -> Function/1
  Function/1 -> y(1)/1

== system VirtualADAPT/VirtualADAPTv1/PowerDistribution/ACLoadBank2/L2E/LGT7/LightBulb1/TemperatureModel/ModPs ==
  u(1) : Inport
  Function : Fcn  Expr=u(1)
  Mux : Mux  Inputs=1
  y(1) : Outport
  wires:
  u(1)/1 -> Mux/1
  Mux/1 -> Function/1
  Function/1 -> y(1)/1

== system VirtualADAPT/VirtualADAPTv1/PowerDistribution/ACLoadBank2/L2E/LGT7/LightBulb1/TemperatureModel/OJ ==
  u(1) : Inport
  u(2) : Inport  Port=2
  u(3) : Inport  Port=3
  Adder : Sum  SaturateOnIntegerOverflow=on  InputSameDT=on  OutDataTypeStr=Inherit: Same as first input
  Gain : Gain  SaturateOnIntegerOverflow=on  OutDataTypeStr=Inherit: Same as input  ParamDataTypeStr=Inherit: Same as input
  y(1) : Outport
  y(2) : Outport  Port=2
  y(3) : Outport  Port=3
  O : Outport  Port=4
  wires:
  Adder/1 -> Gain/1
  u(2)/1 -> y(1)/1, y(3)/1, O/1
  u(1)/1 -> Adder/1
  Gain/1 -> y(2)/1
  u(3)/1 -> Adder/2

== system VirtualADAPT/VirtualADAPTv1/PowerDistribution/ACLoadBank2/L2E/LGT7/LightBulb1/TemperatureModel/Rtheta ==
  In : Inport
  Bond : Constant  Value=2
  Computing R : Subsystem
  Fault Profile : Constant  Value=FP
  JuncDB : Constant  Value=2
  Junction Type : Constant
  Ramp : simulink/Sources/Ramp  slope=FM  start=FT  X0=R
  Resistor Value : Constant  Value=R
  Step : Step  Time=FT  Before=R  After=(1 + FM)*R  SampleTime=0
  Out : Outport
  R : Outport  Port=2
  wires:
  Computing R/1(Out1) -> Out/1
  In/1 -> Computing R/1(In1)
  Resistor Value/1 -> Computing R/2(RNominal)
  Fault Profile/1 -> Computing R/3(Fault Profile)
  Step/1 -> Computing R/4(Step)
  Ramp/1 -> Computing R/5(Ramp)
  JuncDB/1 -> Computing R/6(JuncDB)
  Bond/1 -> Computing R/7(Bond)
  Junction Type/1 -> Computing R/8(Junction Type)
  Computing R/2(Out2) -> R/1

== system VirtualADAPT/VirtualADAPTv1/PowerDistribution/ACLoadBank2/L2E/LGT7/LightBulb1/TemperatureModel/SfPs ==
  E : Inport
  Sf : Inport  Port=2
  Bias : Step  Time=FT  After=FM  SampleTime=0
  Fault Inject : Subsystem
  Fault Profile : Constant  Value=FP
  Mult2 : Product  SaturateOnIntegerOverflow=on  RndMeth=Zero  InputSameDT=on  OutDataTypeStr=Inherit: Same as first input
  Multiply : Product  SaturateOnIntegerOverflow=on  RndMeth=Zero  InputSameDT=on  OutDataTypeStr=Inherit: Same as first input
  Ramp : simulink/Sources/Ramp  slope=FM  start=FT  X0=0
  Step : Step  Time=FT  Before=1  After=(1 + FM)  SampleTime=0
  StuckAt : Step  Time=FT  Before=1  After=0  SampleTime=0
  StuckAt2 : Step  Time=FT  After=FM  SampleTime=0
  Sum : Sum  SaturateOnIntegerOverflow=on  InputSameDT=on  OutDataTypeStr=Inherit: Same as first input
  Sum2 : Sum  SaturateOnIntegerOverflow=on  InputSameDT=on  OutDataTypeStr=Inherit: Same as first input
  Sum3 : Sum  SaturateOnIntegerOverflow=on  InputSameDT=on  OutDataTypeStr=Inherit: Same as first input
  F : Outport
  wires:
  Fault Inject/1(Out1) -> F/1
  Sf/1 -> Fault Inject/1(Nominal), Multiply/1, Sum/1, Sum2/1, Mult2/1
  Fault Profile/1 -> Fault Inject/2(Fault Profile)
  Step/1 -> Multiply/2
  Ramp/1 -> Sum/2
  Multiply/1 -> Fault Inject/3(Step)
  Sum/1 -> Fault Inject/4(Ramp)
  Bias/1 -> Sum2/2
  Sum2/1 -> Fault Inject/5(Bias)
  StuckAt/1 -> Mult2/2
  Mult2/1 -> Sum3/1
  StuckAt2/1 -> Sum3/2
  Sum3/1 -> Fault Inject/6(stuckAt)

== system VirtualADAPT/VirtualADAPTv1/PowerDistribution/ACLoadBank2/L2E/LGT7/LightBulb1/TemperatureModel/theta ==
  u(1) : Inport
  u(2) : Inport  Port=2
  u(3) : Inport  Port=3
  Adder : Sum  Inputs=+-  SaturateOnIntegerOverflow=on  InputSameDT=on  OutDataTypeStr=Inherit: Same as first input
  Gain : Gain  SaturateOnIntegerOverflow=on  OutDataTypeStr=Inherit: Same as input  ParamDataTypeStr=Inherit: Same as input
  y(1) : Outport
  y(2) : Outport  Port=2
  y(3) : Outport  Port=3
  O : Outport  Port=4
  wires:
  Adder/1 -> Gain/1
  u(3)/1 -> y(1)/1, y(2)/1, O/1
  u(1)/1 -> Adder/1
  u(2)/1 -> Adder/2
  Gain/1 -> y(3)/1

== system VirtualADAPT/VirtualADAPTv1/PowerDistribution/ACLoadBank2/L2E/LGT7/LightBulb2/R/Computing R ==
  In1 : Inport
  RNominal : Inport  Port=2
  Fault Profile : Inport  Port=3
  Step : Inport  Port=4
  Ramp : Inport  Port=5
  JuncDB : Inport  Port=6
  Bond : Inport  Port=7
  Junction Type : Inport  Port=8
  CalcCausality : Fcn  Expr=(u(1) == u(2) && u(3) ==1) || (u(1) !=u(2) && u(3) == 0)
  CalcOutput : Fcn  Expr=(u(1)*u(2)^u(3))
  MinusOne : Constant  Value=-1
  Mux1 : Mux  Inputs=3
  Mux2 : Mux  Inputs=3
  One : Constant
  Switch1 : MultiPortSwitch  SaturateOnIntegerOverflow=on  InputSameDT=on
  Switch2 : MultiPortSwitch  DataPortOrder=Zero-based contiguous  Inputs=2  zeroidx=on  SaturateOnIntegerOverflow=on  InputSameDT=on
  Out1 : Outport
  Out2 : Outport  Port=2
  wires:
  In1/1 -> Mux2/1
  Fault Profile/1 -> Switch1/1
  RNominal/1 -> Switch1/2
  Step/1 -> Switch1/3
  Ramp/1 -> Switch1/4
  Switch1/1 -> Out2/1, Mux2/2
  JuncDB/1 -> Mux1/1
  Bond/1 -> Mux1/2
  Junction Type/1 -> Mux1/3
  Mux1/1 -> CalcCausality/1
  CalcCausality/1 -> Switch2/1
  One/1 -> Switch2/2
  MinusOne/1 -> Switch2/3
  Switch2/1 -> Mux2/3
  Mux2/1 -> CalcOutput/1
  CalcOutput/1 -> Out1/1

== system VirtualADAPT/VirtualADAPTv1/PowerDistribution/ACLoadBank2/L2E/LGT7/LightBulb2/TemperatureModel/AmbTemp ==
  F : Inport
  Se : Inport  Port=2
  Bias : Step  Time=FT  After=FM  SampleTime=0
  Fault Inject : Subsystem
  Fault Profile : Constant  Value=FP
  Mult2 : Product  SaturateOnIntegerOverflow=on  RndMeth=Zero  InputSameDT=on  OutDataTypeStr=Inherit: Same as first input
  Multiply : Product  SaturateOnIntegerOverflow=on  RndMeth=Zero  InputSameDT=on  OutDataTypeStr=Inherit: Same as first input
  Ramp : simulink/Sources/Ramp  slope=FM  start=FT  X0=0
  Step : Step  Time=FT  Before=1  After=(1 + FM)  SampleTime=0
  StuckAt : Step  Time=FT  Before=1  After=0  SampleTime=0
  StuckAt2 : Step  Time=FT  After=FM  SampleTime=0
  Sum : Sum  SaturateOnIntegerOverflow=on  InputSameDT=on  OutDataTypeStr=Inherit: Same as first input
  Sum2 : Sum  SaturateOnIntegerOverflow=on  InputSameDT=on  OutDataTypeStr=Inherit: Same as first input
  Sum3 : Sum  SaturateOnIntegerOverflow=on  InputSameDT=on  OutDataTypeStr=Inherit: Same as first input
  E : Outport
  wires:
  Fault Inject/1(Out1) -> E/1
  Se/1 -> Fault Inject/1(Nominal), Multiply/1, Sum/1, Sum2/1, Mult2/1
  Fault Profile/1 -> Fault Inject/2(Fault Profile)
  Step/1 -> Multiply/2
  Ramp/1 -> Sum/2
  Multiply/1 -> Fault Inject/3(Step)
  Sum/1 -> Fault Inject/4(Ramp)
  Bias/1 -> Sum2/2
  Sum2/1 -> Fault Inject/5(Bias)
  StuckAt/1 -> Mult2/2
  Mult2/1 -> Sum3/1
  StuckAt2/1 -> Sum3/2
  Sum3/1 -> Fault Inject/6(stuckAt)

== system VirtualADAPT/VirtualADAPTv1/PowerDistribution/ACLoadBank2/L2E/LGT7/LightBulb2/TemperatureModel/Ctheta ==
  F : Inport
  Capacitance : Constant  Value=C
  Data Type Conversion : DataTypeConversion  OutDataTypeStr=boolean
  Detect Change : simulink/Logic and Bit Operations/Detect Change  vinit=0  InputProcessing=Inherited  OutDataTypeStr=uint8
  Fault Inject : Subsystem
  Fault Profile : Constant  Value=FP
  InitialCondition : Constant  Value=IC
  Integrator : Integrator  ExternalReset=either  InitialConditionSource=external
  Product1 : Product  SaturateOnIntegerOverflow=on  RndMeth=Zero  InputSameDT=on  OutDataTypeStr=Inherit: Same as first input
  Product2 : Product  SaturateOnIntegerOverflow=on  RndMeth=Zero  InputSameDT=on  OutDataTypeStr=Inherit: Same as first input
  Ramp : simulink/Sources/Ramp  slope=FM  start=FT  X0=C
  Reciprocal : Math  Operator=reciprocal  SaturateOnIntegerOverflow=on
  Step : Step  Time=FT  Before=C  After=(1 + FM)*C  SampleTime=0
  E : Outport
  C : Outport  Port=2
  q : Outport  Port=3
  wires:
  F/1 -> Integrator/1
  InitialCondition/1 -> Product1/1, Detect Change/1
  Capacitance/1 -> Fault Inject/1(Nominal), Product1/2
  Product1/1 -> Integrator/3
  Integrator/1 -> Product2/1, q/1
  Fault Inject/1(Out1) -> Reciprocal/1, C/1
  Reciprocal/1 -> Product2/2
  Fault Profile/1 -> Fault Inject/2(Fault Profile)
  Step/1 -> Fault Inject/3(Step)
  Ramp/1 -> Fault Inject/4(Ramp)
  Product2/1 -> E/1
  Detect Change/1 -> Data Type Conversion/1
  Data Type Conversion/1 -> Integrator/2

== system VirtualADAPT/VirtualADAPTv1/PowerDistribution/ACLoadBank2/L2E/LGT7/LightBulb2/TemperatureModel/ModAmbTemp ==
  u(1) : Inport
  Function : Fcn  Expr=-u(1)
  Mux : Mux  Inputs=1
  y(1) : Outport
  wires:
  u(1)/1 -> Mux/1
  Mux/1 -> Function/1
  Function/1 -> y(1)/1

== system VirtualADAPT/VirtualADAPTv1/PowerDistribution/ACLoadBank2/L2E/LGT7/LightBulb2/TemperatureModel/ModPs ==
  u(1) : Inport
  Function : Fcn  Expr=u(1)
  Mux : Mux  Inputs=1
  y(1) : Outport
  wires:
  u(1)/1 -> Mux/1
  Mux/1 -> Function/1
  Function/1 -> y(1)/1

== system VirtualADAPT/VirtualADAPTv1/PowerDistribution/ACLoadBank2/L2E/LGT7/LightBulb2/TemperatureModel/OJ ==
  u(1) : Inport
  u(2) : Inport  Port=2
  u(3) : Inport  Port=3
  Adder : Sum  SaturateOnIntegerOverflow=on  InputSameDT=on  OutDataTypeStr=Inherit: Same as first input
  Gain : Gain  SaturateOnIntegerOverflow=on  OutDataTypeStr=Inherit: Same as input  ParamDataTypeStr=Inherit: Same as input
  y(1) : Outport
  y(2) : Outport  Port=2
  y(3) : Outport  Port=3
  O : Outport  Port=4
  wires:
  Adder/1 -> Gain/1
  u(2)/1 -> y(1)/1, y(3)/1, O/1
  u(1)/1 -> Adder/1
  Gain/1 -> y(2)/1
  u(3)/1 -> Adder/2

== system VirtualADAPT/VirtualADAPTv1/PowerDistribution/ACLoadBank2/L2E/LGT7/LightBulb2/TemperatureModel/Rtheta ==
  In : Inport
  Bond : Constant  Value=2
  Computing R : Subsystem
  Fault Profile : Constant  Value=FP
  JuncDB : Constant  Value=2
  Junction Type : Constant
  Ramp : simulink/Sources/Ramp  slope=FM  start=FT  X0=R
  Resistor Value : Constant  Value=R
  Step : Step  Time=FT  Before=R  After=(1 + FM)*R  SampleTime=0
  Out : Outport
  R : Outport  Port=2
  wires:
  Computing R/1(Out1) -> Out/1
  In/1 -> Computing R/1(In1)
  Resistor Value/1 -> Computing R/2(RNominal)
  Fault Profile/1 -> Computing R/3(Fault Profile)
  Step/1 -> Computing R/4(Step)
  Ramp/1 -> Computing R/5(Ramp)
  JuncDB/1 -> Computing R/6(JuncDB)
  Bond/1 -> Computing R/7(Bond)
  Junction Type/1 -> Computing R/8(Junction Type)
  Computing R/2(Out2) -> R/1

== system VirtualADAPT/VirtualADAPTv1/PowerDistribution/ACLoadBank2/L2E/LGT7/LightBulb2/TemperatureModel/SfPs ==
  E : Inport
  Sf : Inport  Port=2
  Bias : Step  Time=FT  After=FM  SampleTime=0
  Fault Inject : Subsystem
  Fault Profile : Constant  Value=FP
  Mult2 : Product  SaturateOnIntegerOverflow=on  RndMeth=Zero  InputSameDT=on  OutDataTypeStr=Inherit: Same as first input
  Multiply : Product  SaturateOnIntegerOverflow=on  RndMeth=Zero  InputSameDT=on  OutDataTypeStr=Inherit: Same as first input
  Ramp : simulink/Sources/Ramp  slope=FM  start=FT  X0=0
  Step : Step  Time=FT  Before=1  After=(1 + FM)  SampleTime=0
  StuckAt : Step  Time=FT  Before=1  After=0  SampleTime=0
  StuckAt2 : Step  Time=FT  After=FM  SampleTime=0
  Sum : Sum  SaturateOnIntegerOverflow=on  InputSameDT=on  OutDataTypeStr=Inherit: Same as first input
  Sum2 : Sum  SaturateOnIntegerOverflow=on  InputSameDT=on  OutDataTypeStr=Inherit: Same as first input
  Sum3 : Sum  SaturateOnIntegerOverflow=on  InputSameDT=on  OutDataTypeStr=Inherit: Same as first input
  F : Outport
  wires:
  Fault Inject/1(Out1) -> F/1
  Sf/1 -> Fault Inject/1(Nominal), Multiply/1, Sum/1, Sum2/1, Mult2/1
  Fault Profile/1 -> Fault Inject/2(Fault Profile)
  Step/1 -> Multiply/2
  Ramp/1 -> Sum/2
  Multiply/1 -> Fault Inject/3(Step)
  Sum/1 -> Fault Inject/4(Ramp)
  Bias/1 -> Sum2/2
  Sum2/1 -> Fault Inject/5(Bias)
  StuckAt/1 -> Mult2/2
  Mult2/1 -> Sum3/1
  StuckAt2/1 -> Sum3/2
  Sum3/1 -> Fault Inject/6(stuckAt)

== system VirtualADAPT/VirtualADAPTv1/PowerDistribution/ACLoadBank2/L2E/LGT7/LightBulb2/TemperatureModel/theta ==
  u(1) : Inport
  u(2) : Inport  Port=2
  u(3) : Inport  Port=3
  Adder : Sum  Inputs=+-  SaturateOnIntegerOverflow=on  InputSameDT=on  OutDataTypeStr=Inherit: Same as first input
  Gain : Gain  SaturateOnIntegerOverflow=on  OutDataTypeStr=Inherit: Same as input  ParamDataTypeStr=Inherit: Same as input
  y(1) : Outport
  y(2) : Outport  Port=2
  y(3) : Outport  Port=3
  O : Outport  Port=4
  wires:
  Adder/1 -> Gain/1
  u(3)/1 -> y(1)/1, y(2)/1, O/1
  u(1)/1 -> Adder/1
  u(2)/1 -> Adder/2
  Gain/1 -> y(3)/1

== system VirtualADAPT/VirtualADAPTv1/PowerDistribution/ACLoadBank2/L2E/LGT7/LightBulb3/R/Computing R ==
  In1 : Inport
  RNominal : Inport  Port=2
  Fault Profile : Inport  Port=3
  Step : Inport  Port=4
  Ramp : Inport  Port=5
  JuncDB : Inport  Port=6
  Bond : Inport  Port=7
  Junction Type : Inport  Port=8
  CalcCausality : Fcn  Expr=(u(1) == u(2) && u(3) ==1) || (u(1) !=u(2) && u(3) == 0)
  CalcOutput : Fcn  Expr=(u(1)*u(2)^u(3))
  MinusOne : Constant  Value=-1
  Mux1 : Mux  Inputs=3
  Mux2 : Mux  Inputs=3
  One : Constant
  Switch1 : MultiPortSwitch  SaturateOnIntegerOverflow=on  InputSameDT=on
  Switch2 : MultiPortSwitch  DataPortOrder=Zero-based contiguous  Inputs=2  zeroidx=on  SaturateOnIntegerOverflow=on  InputSameDT=on
  Out1 : Outport
  Out2 : Outport  Port=2
  wires:
  In1/1 -> Mux2/1
  Fault Profile/1 -> Switch1/1
  RNominal/1 -> Switch1/2
  Step/1 -> Switch1/3
  Ramp/1 -> Switch1/4
  Switch1/1 -> Out2/1, Mux2/2
  JuncDB/1 -> Mux1/1
  Bond/1 -> Mux1/2
  Junction Type/1 -> Mux1/3
  Mux1/1 -> CalcCausality/1
  CalcCausality/1 -> Switch2/1
  One/1 -> Switch2/2
  MinusOne/1 -> Switch2/3
  Switch2/1 -> Mux2/3
  Mux2/1 -> CalcOutput/1
  CalcOutput/1 -> Out1/1

== system VirtualADAPT/VirtualADAPTv1/PowerDistribution/ACLoadBank2/L2E/LGT7/LightBulb3/TemperatureModel/AmbTemp ==
  F : Inport
  Se : Inport  Port=2
  Bias : Step  Time=FT  After=FM  SampleTime=0
  Fault Inject : Subsystem
  Fault Profile : Constant  Value=FP
  Mult2 : Product  SaturateOnIntegerOverflow=on  RndMeth=Zero  InputSameDT=on  OutDataTypeStr=Inherit: Same as first input
  Multiply : Product  SaturateOnIntegerOverflow=on  RndMeth=Zero  InputSameDT=on  OutDataTypeStr=Inherit: Same as first input
  Ramp : simulink/Sources/Ramp  slope=FM  start=FT  X0=0
  Step : Step  Time=FT  Before=1  After=(1 + FM)  SampleTime=0
  StuckAt : Step  Time=FT  Before=1  After=0  SampleTime=0
  StuckAt2 : Step  Time=FT  After=FM  SampleTime=0
  Sum : Sum  SaturateOnIntegerOverflow=on  InputSameDT=on  OutDataTypeStr=Inherit: Same as first input
  Sum2 : Sum  SaturateOnIntegerOverflow=on  InputSameDT=on  OutDataTypeStr=Inherit: Same as first input
  Sum3 : Sum  SaturateOnIntegerOverflow=on  InputSameDT=on  OutDataTypeStr=Inherit: Same as first input
  E : Outport
  wires:
  Fault Inject/1(Out1) -> E/1
  Se/1 -> Fault Inject/1(Nominal), Multiply/1, Sum/1, Sum2/1, Mult2/1
  Fault Profile/1 -> Fault Inject/2(Fault Profile)
  Step/1 -> Multiply/2
  Ramp/1 -> Sum/2
  Multiply/1 -> Fault Inject/3(Step)
  Sum/1 -> Fault Inject/4(Ramp)
  Bias/1 -> Sum2/2
  Sum2/1 -> Fault Inject/5(Bias)
  StuckAt/1 -> Mult2/2
  Mult2/1 -> Sum3/1
  StuckAt2/1 -> Sum3/2
  Sum3/1 -> Fault Inject/6(stuckAt)

== system VirtualADAPT/VirtualADAPTv1/PowerDistribution/ACLoadBank2/L2E/LGT7/LightBulb3/TemperatureModel/Ctheta ==
  F : Inport
  Capacitance : Constant  Value=C
  Data Type Conversion : DataTypeConversion  OutDataTypeStr=boolean
  Detect Change : simulink/Logic and Bit Operations/Detect Change  vinit=0  InputProcessing=Inherited  OutDataTypeStr=uint8
  Fault Inject : Subsystem
  Fault Profile : Constant  Value=FP
  InitialCondition : Constant  Value=IC
  Integrator : Integrator  ExternalReset=either  InitialConditionSource=external
  Product1 : Product  SaturateOnIntegerOverflow=on  RndMeth=Zero  InputSameDT=on  OutDataTypeStr=Inherit: Same as first input
  Product2 : Product  SaturateOnIntegerOverflow=on  RndMeth=Zero  InputSameDT=on  OutDataTypeStr=Inherit: Same as first input
  Ramp : simulink/Sources/Ramp  slope=FM  start=FT  X0=C
  Reciprocal : Math  Operator=reciprocal  SaturateOnIntegerOverflow=on
  Step : Step  Time=FT  Before=C  After=(1 + FM)*C  SampleTime=0
  E : Outport
  C : Outport  Port=2
  q : Outport  Port=3
  wires:
  F/1 -> Integrator/1
  InitialCondition/1 -> Product1/1, Detect Change/1
  Capacitance/1 -> Fault Inject/1(Nominal), Product1/2
  Product1/1 -> Integrator/3
  Integrator/1 -> Product2/1, q/1
  Fault Inject/1(Out1) -> Reciprocal/1, C/1
  Reciprocal/1 -> Product2/2
  Fault Profile/1 -> Fault Inject/2(Fault Profile)
  Step/1 -> Fault Inject/3(Step)
  Ramp/1 -> Fault Inject/4(Ramp)
  Product2/1 -> E/1
  Detect Change/1 -> Data Type Conversion/1
  Data Type Conversion/1 -> Integrator/2

== system VirtualADAPT/VirtualADAPTv1/PowerDistribution/ACLoadBank2/L2E/LGT7/LightBulb3/TemperatureModel/ModAmbTemp ==
  u(1) : Inport
  Function : Fcn  Expr=-u(1)
  Mux : Mux  Inputs=1
  y(1) : Outport
  wires:
  u(1)/1 -> Mux/1
  Mux/1 -> Function/1
  Function/1 -> y(1)/1

== system VirtualADAPT/VirtualADAPTv1/PowerDistribution/ACLoadBank2/L2E/LGT7/LightBulb3/TemperatureModel/ModPs ==
  u(1) : Inport
  Function : Fcn  Expr=u(1)
  Mux : Mux  Inputs=1
  y(1) : Outport
  wires:
  u(1)/1 -> Mux/1
  Mux/1 -> Function/1
  Function/1 -> y(1)/1

== system VirtualADAPT/VirtualADAPTv1/PowerDistribution/ACLoadBank2/L2E/LGT7/LightBulb3/TemperatureModel/OJ ==
  u(1) : Inport
  u(2) : Inport  Port=2
  u(3) : Inport  Port=3
  Adder : Sum  SaturateOnIntegerOverflow=on  InputSameDT=on  OutDataTypeStr=Inherit: Same as first input
  Gain : Gain  SaturateOnIntegerOverflow=on  OutDataTypeStr=Inherit: Same as input  ParamDataTypeStr=Inherit: Same as input
  y(1) : Outport
  y(2) : Outport  Port=2
  y(3) : Outport  Port=3
  O : Outport  Port=4
  wires:
  Adder/1 -> Gain/1
  u(2)/1 -> y(1)/1, y(3)/1, O/1
  u(1)/1 -> Adder/1
  Gain/1 -> y(2)/1
  u(3)/1 -> Adder/2

== system VirtualADAPT/VirtualADAPTv1/PowerDistribution/ACLoadBank2/L2E/LGT7/LightBulb3/TemperatureModel/Rtheta ==
  In : Inport
  Bond : Constant  Value=2
  Computing R : Subsystem
  Fault Profile : Constant  Value=FP
  JuncDB : Constant  Value=2
  Junction Type : Constant
  Ramp : simulink/Sources/Ramp  slope=FM  start=FT  X0=R
  Resistor Value : Constant  Value=R
  Step : Step  Time=FT  Before=R  After=(1 + FM)*R  SampleTime=0
  Out : Outport
  R : Outport  Port=2
  wires:
  Computing R/1(Out1) -> Out/1
  In/1 -> Computing R/1(In1)
  Resistor Value/1 -> Computing R/2(RNominal)
  Fault Profile/1 -> Computing R/3(Fault Profile)
  Step/1 -> Computing R/4(Step)
  Ramp/1 -> Computing R/5(Ramp)
  JuncDB/1 -> Computing R/6(JuncDB)
  Bond/1 -> Computing R/7(Bond)
  Junction Type/1 -> Computing R/8(Junction Type)
  Computing R/2(Out2) -> R/1

== system VirtualADAPT/VirtualADAPTv1/PowerDistribution/ACLoadBank2/L2E/LGT7/LightBulb3/TemperatureModel/SfPs ==
  E : Inport
  Sf : Inport  Port=2
  Bias : Step  Time=FT  After=FM  SampleTime=0
  Fault Inject : Subsystem
  Fault Profile : Constant  Value=FP
  Mult2 : Product  SaturateOnIntegerOverflow=on  RndMeth=Zero  InputSameDT=on  OutDataTypeStr=Inherit: Same as first input
  Multiply : Product  SaturateOnIntegerOverflow=on  RndMeth=Zero  InputSameDT=on  OutDataTypeStr=Inherit: Same as first input
  Ramp : simulink/Sources/Ramp  slope=FM  start=FT  X0=0
  Step : Step  Time=FT  Before=1  After=(1 + FM)  SampleTime=0
  StuckAt : Step  Time=FT  Before=1  After=0  SampleTime=0
  StuckAt2 : Step  Time=FT  After=FM  SampleTime=0
  Sum : Sum  SaturateOnIntegerOverflow=on  InputSameDT=on  OutDataTypeStr=Inherit: Same as first input
  Sum2 : Sum  SaturateOnIntegerOverflow=on  InputSameDT=on  OutDataTypeStr=Inherit: Same as first input
  Sum3 : Sum  SaturateOnIntegerOverflow=on  InputSameDT=on  OutDataTypeStr=Inherit: Same as first input
  F : Outport
  wires:
  Fault Inject/1(Out1) -> F/1
  Sf/1 -> Fault Inject/1(Nominal), Multiply/1, Sum/1, Sum2/1, Mult2/1
  Fault Profile/1 -> Fault Inject/2(Fault Profile)
  Step/1 -> Multiply/2
  Ramp/1 -> Sum/2
  Multiply/1 -> Fault Inject/3(Step)
  Sum/1 -> Fault Inject/4(Ramp)
  Bias/1 -> Sum2/2
  Sum2/1 -> Fault Inject/5(Bias)
  StuckAt/1 -> Mult2/2
  Mult2/1 -> Sum3/1
  StuckAt2/1 -> Sum3/2
  Sum3/1 -> Fault Inject/6(stuckAt)

== system VirtualADAPT/VirtualADAPTv1/PowerDistribution/ACLoadBank2/L2E/LGT7/LightBulb3/TemperatureModel/theta ==
  u(1) : Inport
  u(2) : Inport  Port=2
  u(3) : Inport  Port=3
  Adder : Sum  Inputs=+-  SaturateOnIntegerOverflow=on  InputSameDT=on  OutDataTypeStr=Inherit: Same as first input
  Gain : Gain  SaturateOnIntegerOverflow=on  OutDataTypeStr=Inherit: Same as input  ParamDataTypeStr=Inherit: Same as input
  y(1) : Outport
  y(2) : Outport  Port=2
  y(3) : Outport  Port=3
  O : Outport  Port=4
  wires:
  Adder/1 -> Gain/1
  u(3)/1 -> y(1)/1, y(2)/1, O/1
  u(1)/1 -> Adder/1
  u(2)/1 -> Adder/2
  Gain/1 -> y(3)/1

== system VirtualADAPT/VirtualADAPTv1/PowerDistribution/ACLoadBank1/L1A/LGT6/LightBulb1/TemperatureModel/AmbTemp/Fault Inject ==
  Nominal : Inport
  Fault Profile : Inport  Port=2
  Step : Inport  Port=3
  Ramp : Inport  Port=4
  Bias : Inport  Port=5
  stuckAt : Inport  Port=6
  Switch1 : MultiPortSwitch  Inputs=5  SaturateOnIntegerOverflow=on  InputSameDT=on
  Out1 : Outport
  wires:
  Nominal/1 -> Switch1/2
  Fault Profile/1 -> Switch1/1
  Step/1 -> Switch1/3
  Ramp/1 -> Switch1/4
  Bias/1 -> Switch1/5
  stuckAt/1 -> Switch1/6
  Switch1/1 -> Out1/1

== system VirtualADAPT/VirtualADAPTv1/PowerDistribution/ACLoadBank1/L1A/LGT6/LightBulb1/TemperatureModel/Ctheta/Fault Inject ==
  Nominal : Inport
  Fault Profile : Inport  Port=2
  Step : Inport  Port=3
  Ramp : Inport  Port=4
  Bias : Inport  Port=5
  stuckAt : Inport  Port=6
  Switch1 : MultiPortSwitch  Inputs=5  SaturateOnIntegerOverflow=on  InputSameDT=on
  Out1 : Outport
  wires:
  Nominal/1 -> Switch1/2
  Fault Profile/1 -> Switch1/1
  Step/1 -> Switch1/3
  Ramp/1 -> Switch1/4
  Bias/1 -> Switch1/5
  stuckAt/1 -> Switch1/6
  Switch1/1 -> Out1/1
